simulink model "TestHarness_inf"  (R2016a)
settings: Solver=FixedStepDiscrete  StartTime=0.0  StopTime=120  FixedStep=0.001  RelTol=1e-3
undefined symbols (not defined in the file or in its folder): sigbuilder_block
libraries: simulink x8, gate_V32_2016a_Lib_inf x1, realtime_pacer_lib x1
settings Model: Creator=oelkawok  LastModifiedBy=oelkawok
settings DataIOCC: SaveFormat=StructureWithTime
settings OptimizationCC: DefaultParameterBehavior=Inlined  StateBitsets=on  DataBitsets=on  LifeSpan=1  MaxStackSize=64
settings DebuggingCC: ConsistencyChecking=error  ArrayBoundsChecking=error  SignalInfNanChecking=error  SignalRangeChecking=error  ReadBeforeWriteMsg=EnableAllAsError  WriteAfterWriteMsg=EnableAllAsError  WriteAfterReadMsg=EnableAllAsError  AlgebraicLoopMsg=error  ArtificialAlgebraicLoopMsg=error  BlockPriorityViolationMsg=error  MinStepSizeMsg=error  MultiTaskCondExecSysMsg=none  MultiTaskRateTransMsg=warning  CheckMatrixSingularityMsg=error  ParameterUnderflowMsg=error  ParameterPrecisionLossMsg=error  UnderSpecifiedDataTypeMsg=warning  UnnecessaryDatatypeConvMsg=warning  VectorMatrixConversionMsg=error  SignalLabelMismatchMsg=error  UnconnectedInputMsg=error  UnconnectedOutputMsg=error  UnconnectedLineMsg=error  SFcnCompatibilityMsg=error  UniqueDataStoreMsg=error  BusObjectLabelMismatch=error  RootOutportRequireBusObject=error  ModelReferenceIOMsg=error  ModelReferenceVersionMismatchMessage=error  ModelReferenceIOMismatchMessage=error  ModelReferenceDataLoggingMessage=error  StrictBusMsg=ErrorOnBusTreatedAsVector  SFUnusedDataAndEventsDiag=error  SFUnexpectedBacktrackingDiag=error  SFInvalidInputDataAccessInChartInitDiag=error  SFNoUnconditionalDefaultTransitionDiag=error  SFTransitionOutsideNaturalParentDiag=error  SFUndirectedBroadcastEventsDiag=error  SFTransitionActionBeforeConditionDiag=error  SFOutputUsedAsStateInMooreChartDiag=warning
settings HardwareCC: ProdLongLongMode=on
settings SFSimCC: SimCustomHeaderCode=|
  | //#include "C:\Users\omaro\Desktop\Gate_Model\Arduino\Arduino.h"
  | //#include "Keypad\Keypad.h"
settings RTWCC: SystemTargetFile=ert.tlc  TemplateMakefile=ert_default_tmf  GenerateReport=on  RTWUseSimCustomCode=on  Toolchain=Arduino AVR v1.6.1  BuildConfiguration=Faster Runs  LaunchReport=on
settings RTWCC: CustomHeaderCode=|
  | //#include "C:\Users\omaro\Desktop\Gate_Model\Arduino\Arduino.h"
  | //#include "Keypad\Keypad.h"
settings CodeAppCC: ForceParamTrailComments=on  OperatorAnnotations=on  MATLABSourceComments=on
settings ERTTargetCC: ERTCustomFileBanners=off  SupportContinuousTime=on  SupportNonInlinedSFcns=on  SupportVariableSizeSignals=on  ModelStepFunctionPrototypeControlCompliant=off  RootIOFormat=Structure reference  ERTCustomFileTemplate=example_file_process.tlc

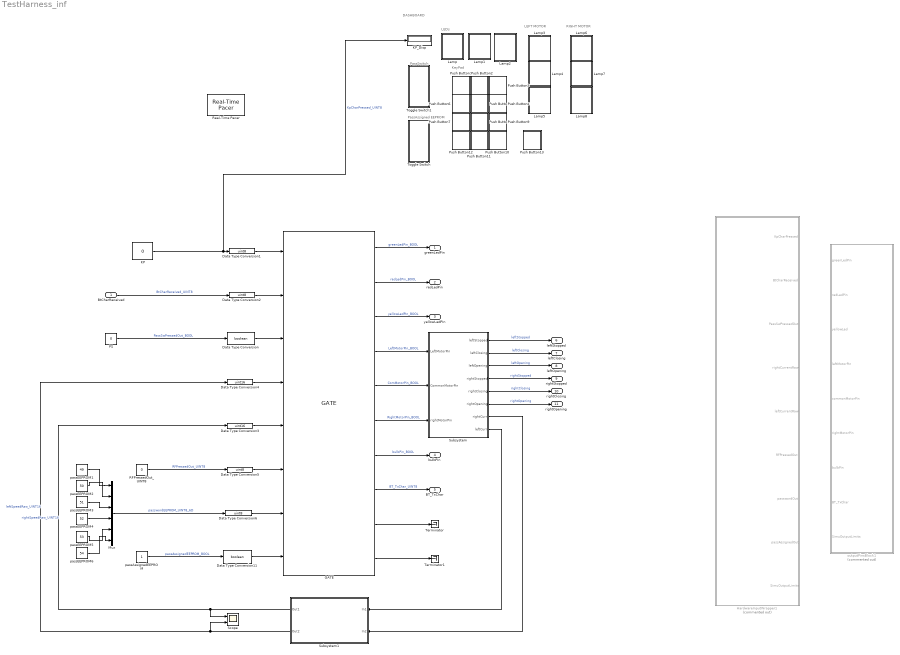
== system TestHarness_inf ==
  note: PassSwitch
  note: PassAssigned
      | EEPROM
  note: KeyPad
  note: DASHBOARD
  note: LEDs
  note: LEFT MOTOR
  note: RIGHT MOTOR
  "BtCharReceived " : Inport
  Data Type Conversion : DataTypeConversion  OutDataTypeStr=boolean
  Data Type Conversion{1..2,5..6} (4 alike) : DataTypeConversion  OutDataTypeStr=uint8
  Data Type Conversion11 : DataTypeConversion  OutDataTypeStr=boolean
  Data Type Conversion3 : DataTypeConversion  OutDataTypeStr=uint16
  Data Type Conversion4 : DataTypeConversion  OutDataTypeStr=uint16
  GATE : gate_V32_2016a_Lib_inf/GATE  VariantControl=Variant1  OverrideUsingVariant=VariantB
  HardwareInputWrapper1 : Subsystem  Commented=on  Variant=on  VariantControl=Variant1  OverrideUsingVariant=MIL_VariantB
  KP : Constant  Value=0  SampleTime=-1
  KP_Disp : Display
      settings: Format=decimal (Stored Integer)
  Lamp : Subsystem  DialogController=hmiCreateDDGDialog  IsWebBlock=on  mask(MWDashboardBlock){webBlockId=dfeecf7f-6466-4b86-8ae3-9a987e31c94e, webBlockType=lamp, ShowInLibBrowser=on, ShowInitialText=on, HMISrcModelName=TestHarness_inf}
      mask prompts: webBlockType "WebBlock Type"; HMISrcModelName "Source Model"
      mask Help: helpview([docroot '/mapfiles/simulink.map'], 'hmi_lamp')
      mask Display:
      | if strcmp(get_param(bdroot(gcb),'BlockDiagramType'), 'library')
      |     image([(matlabroot) '/toolbox/simulink/hmi/icons/Lamp.png']);
      | end
  Lamp1 : Subsystem  DialogController=hmiCreateDDGDialog  IsWebBlock=on  mask(MWDashboardBlock){webBlockId=77b78638-3987-4679-812f-402e2e1d2cbf, webBlockType=lamp, ShowInLibBrowser=on, ShowInitialText=on, HMISrcModelName=TestHarness_inf}
      mask prompts: webBlockType "WebBlock Type"; HMISrcModelName "Source Model"
      mask Help: helpview([docroot '/mapfiles/simulink.map'], 'hmi_lamp')
      mask Display:
      | if strcmp(get_param(bdroot(gcb),'BlockDiagramType'), 'library')
      |     image([(matlabroot) '/toolbox/simulink/hmi/icons/Lamp.png']);
      | end
  Lamp2 : Subsystem  DialogController=hmiCreateDDGDialog  IsWebBlock=on  mask(MWDashboardBlock){webBlockId=16a95052-e057-46e6-b8f2-472c9f701692, webBlockType=lamp, ShowInLibBrowser=on, ShowInitialText=on, HMISrcModelName=TestHarness_inf}
      mask prompts: webBlockType "WebBlock Type"; HMISrcModelName "Source Model"
      mask Help: helpview([docroot '/mapfiles/simulink.map'], 'hmi_lamp')
      mask Display:
      | if strcmp(get_param(bdroot(gcb),'BlockDiagramType'), 'library')
      |     image([(matlabroot) '/toolbox/simulink/hmi/icons/Lamp.png']);
      | end
  Lamp3 : Subsystem  DialogController=hmiCreateDDGDialog  IsWebBlock=on  mask(MWDashboardBlock){webBlockId=c8f1e96f-8ae5-4205-bff4-16b6e81f721f, webBlockType=lamp, ShowInLibBrowser=on, ShowInitialText=on, HMISrcModelName=TestHarness_inf}
      mask prompts: webBlockType "WebBlock Type"; HMISrcModelName "Source Model"
      mask Help: helpview([docroot '/mapfiles/simulink.map'], 'hmi_lamp')
      mask Display:
      | if strcmp(get_param(bdroot(gcb),'BlockDiagramType'), 'library')
      |     image([(matlabroot) '/toolbox/simulink/hmi/icons/Lamp.png']);
      | end
  Lamp4 : Subsystem  DialogController=hmiCreateDDGDialog  IsWebBlock=on  mask(MWDashboardBlock){webBlockId=9178fee7-4670-4670-9e55-d638f178c070, webBlockType=lamp, ShowInLibBrowser=on, ShowInitialText=on, HMISrcModelName=TestHarness_inf}
      mask prompts: webBlockType "WebBlock Type"; HMISrcModelName "Source Model"
      mask Help: helpview([docroot '/mapfiles/simulink.map'], 'hmi_lamp')
      mask Display:
      | if strcmp(get_param(bdroot(gcb),'BlockDiagramType'), 'library')
      |     image([(matlabroot) '/toolbox/simulink/hmi/icons/Lamp.png']);
      | end
  Lamp5 : Subsystem  DialogController=hmiCreateDDGDialog  IsWebBlock=on  mask(MWDashboardBlock){webBlockId=d643292f-cf1e-4cb5-b48c-b958215c0bd7, webBlockType=lamp, ShowInLibBrowser=on, ShowInitialText=on, HMISrcModelName=TestHarness_inf}
      mask prompts: webBlockType "WebBlock Type"; HMISrcModelName "Source Model"
      mask Help: helpview([docroot '/mapfiles/simulink.map'], 'hmi_lamp')
      mask Display:
      | if strcmp(get_param(bdroot(gcb),'BlockDiagramType'), 'library')
      |     image([(matlabroot) '/toolbox/simulink/hmi/icons/Lamp.png']);
      | end
  Lamp6 : Subsystem  DialogController=hmiCreateDDGDialog  IsWebBlock=on  mask(MWDashboardBlock){webBlockId=6bb08dff-de60-4262-bf7f-c7f88bbee20a, webBlockType=lamp, ShowInLibBrowser=on, ShowInitialText=on, HMISrcModelName=TestHarness_inf}
      mask prompts: webBlockType "WebBlock Type"; HMISrcModelName "Source Model"
      mask Help: helpview([docroot '/mapfiles/simulink.map'], 'hmi_lamp')
      mask Display:
      | if strcmp(get_param(bdroot(gcb),'BlockDiagramType'), 'library')
      |     image([(matlabroot) '/toolbox/simulink/hmi/icons/Lamp.png']);
      | end
  Lamp7 : Subsystem  DialogController=hmiCreateDDGDialog  IsWebBlock=on  mask(MWDashboardBlock){webBlockId=b5666a4d-f33d-4bf5-ba64-e318e21455ef, webBlockType=lamp, ShowInLibBrowser=on, ShowInitialText=on, HMISrcModelName=TestHarness_inf}
      mask prompts: webBlockType "WebBlock Type"; HMISrcModelName "Source Model"
      mask Help: helpview([docroot '/mapfiles/simulink.map'], 'hmi_lamp')
      mask Display:
      | if strcmp(get_param(bdroot(gcb),'BlockDiagramType'), 'library')
      |     image([(matlabroot) '/toolbox/simulink/hmi/icons/Lamp.png']);
      | end
  Lamp8 : Subsystem  DialogController=hmiCreateDDGDialog  IsWebBlock=on  mask(MWDashboardBlock){webBlockId=b7bdd2c5-6b26-4275-9419-c5f65add7689, webBlockType=lamp, ShowInLibBrowser=on, ShowInitialText=on, HMISrcModelName=TestHarness_inf}
      mask prompts: webBlockType "WebBlock Type"; HMISrcModelName "Source Model"
      mask Help: helpview([docroot '/mapfiles/simulink.map'], 'hmi_lamp')
      mask Display:
      | if strcmp(get_param(bdroot(gcb),'BlockDiagramType'), 'library')
      |     image([(matlabroot) '/toolbox/simulink/hmi/icons/Lamp.png']);
      | end
  Mux : Mux  Inputs=6
  PS : Constant  Value=0
  Push Button1 : Subsystem  DialogController=hmiCreateDDGDialog  IsWebBlock=on  mask(MWDashboardBlock){webBlockId=efb186e0-7ac7-42d4-9545-c8cda7e6d6ff, webBlockType=pushbutton, ShowInLibBrwoser=on, ShowInitialText=on, HMISrcModelName=TestHarness_inf}
      mask prompts: webBlockId "WebBlock Id"; webBlockType "WebBlock Type"; HMISrcModelName "Source Model"
      mask Help: helpview([docroot '/mapfiles/simulink.map'], 'hmi_push_button')
      mask Display:
      | model = bdroot(gcb);
      | if strcmp(get_param(model,'BlockDiagramType'), 'library')
      |     image([(matlabroot) '/toolbox/simulink/hmi/icons/PushButton.png']);
      | end
  Push Button10 : Subsystem  DialogController=hmiCreateDDGDialog  IsWebBlock=on  mask(MWDashboardBlock){webBlockId=a72d1c9d-87a6-4091-a506-e1108b8799d8, webBlockType=pushbutton, ShowInLibBrwoser=on, ShowInitialText=on, HMISrcModelName=TestHarness_inf}
      mask prompts: webBlockId "WebBlock Id"; webBlockType "WebBlock Type"; HMISrcModelName "Source Model"
      mask Help: helpview([docroot '/mapfiles/simulink.map'], 'hmi_push_button')
      mask Display:
      | model = bdroot(gcb);
      | if strcmp(get_param(model,'BlockDiagramType'), 'library')
      |     image([(matlabroot) '/toolbox/simulink/hmi/icons/PushButton.png']);
      | end
  Push Button11 : Subsystem  DialogController=hmiCreateDDGDialog  IsWebBlock=on  mask(MWDashboardBlock){webBlockId=d494c8aa-0474-4b50-982c-5f796114d678, webBlockType=pushbutton, ShowInLibBrwoser=on, ShowInitialText=on, HMISrcModelName=TestHarness_inf}
      mask prompts: webBlockId "WebBlock Id"; webBlockType "WebBlock Type"; HMISrcModelName "Source Model"
      mask Help: helpview([docroot '/mapfiles/simulink.map'], 'hmi_push_button')
      mask Display:
      | model = bdroot(gcb);
      | if strcmp(get_param(model,'BlockDiagramType'), 'library')
      |     image([(matlabroot) '/toolbox/simulink/hmi/icons/PushButton.png']);
      | end
  Push Button12 : Subsystem  DialogController=hmiCreateDDGDialog  IsWebBlock=on  mask(MWDashboardBlock){webBlockId=f33ddd79-ca68-49fe-b4b6-aee4b307d83a, webBlockType=pushbutton, ShowInLibBrwoser=on, ShowInitialText=on, HMISrcModelName=TestHarness_inf}
      mask prompts: webBlockId "WebBlock Id"; webBlockType "WebBlock Type"; HMISrcModelName "Source Model"
      mask Help: helpview([docroot '/mapfiles/simulink.map'], 'hmi_push_button')
      mask Display:
      | model = bdroot(gcb);
      | if strcmp(get_param(model,'BlockDiagramType'), 'library')
      |     image([(matlabroot) '/toolbox/simulink/hmi/icons/PushButton.png']);
      | end
  Push Button13 : Subsystem  DialogController=hmiCreateDDGDialog  IsWebBlock=on  mask(MWDashboardBlock){webBlockId=e5fe72a6-0ccb-4320-95ea-2ffa7a798bea, webBlockType=pushbutton, ShowInLibBrwoser=on, ShowInitialText=on, HMISrcModelName=TestHarness_inf}
      mask prompts: webBlockId "WebBlock Id"; webBlockType "WebBlock Type"; HMISrcModelName "Source Model"
      mask Help: helpview([docroot '/mapfiles/simulink.map'], 'hmi_push_button')
      mask Display:
      | model = bdroot(gcb);
      | if strcmp(get_param(model,'BlockDiagramType'), 'library')
      |     image([(matlabroot) '/toolbox/simulink/hmi/icons/PushButton.png']);
      | end
  Push Button2 : Subsystem  DialogController=hmiCreateDDGDialog  IsWebBlock=on  mask(MWDashboardBlock){webBlockId=fffe111a-15fb-434e-8e7d-6c74e690b8ec, webBlockType=pushbutton, ShowInLibBrwoser=on, ShowInitialText=on, HMISrcModelName=TestHarness_inf}
      mask prompts: webBlockId "WebBlock Id"; webBlockType "WebBlock Type"; HMISrcModelName "Source Model"
      mask Help: helpview([docroot '/mapfiles/simulink.map'], 'hmi_push_button')
      mask Display:
      | model = bdroot(gcb);
      | if strcmp(get_param(model,'BlockDiagramType'), 'library')
      |     image([(matlabroot) '/toolbox/simulink/hmi/icons/PushButton.png']);
      | end
  Push Button3 : Subsystem  DialogController=hmiCreateDDGDialog  IsWebBlock=on  mask(MWDashboardBlock){webBlockId=7a7e2e11-9f3b-43a3-8fe5-ea89bc2462e2, webBlockType=pushbutton, ShowInLibBrwoser=on, ShowInitialText=on, HMISrcModelName=TestHarness_inf}
      mask prompts: webBlockId "WebBlock Id"; webBlockType "WebBlock Type"; HMISrcModelName "Source Model"
      mask Help: helpview([docroot '/mapfiles/simulink.map'], 'hmi_push_button')
      mask Display:
      | model = bdroot(gcb);
      | if strcmp(get_param(model,'BlockDiagramType'), 'library')
      |     image([(matlabroot) '/toolbox/simulink/hmi/icons/PushButton.png']);
      | end
  Push Button4 : Subsystem  DialogController=hmiCreateDDGDialog  IsWebBlock=on  mask(MWDashboardBlock){webBlockId=4e418dfc-3c1d-4c60-a82e-ca2e05426c13, webBlockType=pushbutton, ShowInLibBrwoser=on, ShowInitialText=on, HMISrcModelName=TestHarness_inf}
      mask prompts: webBlockId "WebBlock Id"; webBlockType "WebBlock Type"; HMISrcModelName "Source Model"
      mask Help: helpview([docroot '/mapfiles/simulink.map'], 'hmi_push_button')
      mask Display:
      | model = bdroot(gcb);
      | if strcmp(get_param(model,'BlockDiagramType'), 'library')
      |     image([(matlabroot) '/toolbox/simulink/hmi/icons/PushButton.png']);
      | end
  Push Button5 : Subsystem  DialogController=hmiCreateDDGDialog  IsWebBlock=on  mask(MWDashboardBlock){webBlockId=b94535ab-6966-44e9-8d7e-8f23ab203339, webBlockType=pushbutton, ShowInLibBrwoser=on, ShowInitialText=on, HMISrcModelName=TestHarness_inf}
      mask prompts: webBlockId "WebBlock Id"; webBlockType "WebBlock Type"; HMISrcModelName "Source Model"
      mask Help: helpview([docroot '/mapfiles/simulink.map'], 'hmi_push_button')
      mask Display:
      | model = bdroot(gcb);
      | if strcmp(get_param(model,'BlockDiagramType'), 'library')
      |     image([(matlabroot) '/toolbox/simulink/hmi/icons/PushButton.png']);
      | end
  Push Button6 : Subsystem  DialogController=hmiCreateDDGDialog  IsWebBlock=on  mask(MWDashboardBlock){webBlockId=823970b4-3e8f-4edd-8037-ba652d66eccb, webBlockType=pushbutton, ShowInLibBrwoser=on, ShowInitialText=on, HMISrcModelName=TestHarness_inf}
      mask prompts: webBlockId "WebBlock Id"; webBlockType "WebBlock Type"; HMISrcModelName "Source Model"
      mask Help: helpview([docroot '/mapfiles/simulink.map'], 'hmi_push_button')
      mask Display:
      | model = bdroot(gcb);
      | if strcmp(get_param(model,'BlockDiagramType'), 'library')
      |     image([(matlabroot) '/toolbox/simulink/hmi/icons/PushButton.png']);
      | end
  Push Button7 : Subsystem  DialogController=hmiCreateDDGDialog  IsWebBlock=on  mask(MWDashboardBlock){webBlockId=b1b2ae4e-6aee-480e-a413-dcdbe3967c1c, webBlockType=pushbutton, ShowInLibBrwoser=on, ShowInitialText=on, HMISrcModelName=TestHarness_inf}
      mask prompts: webBlockId "WebBlock Id"; webBlockType "WebBlock Type"; HMISrcModelName "Source Model"
      mask Help: helpview([docroot '/mapfiles/simulink.map'], 'hmi_push_button')
      mask Display:
      | model = bdroot(gcb);
      | if strcmp(get_param(model,'BlockDiagramType'), 'library')
      |     image([(matlabroot) '/toolbox/simulink/hmi/icons/PushButton.png']);
      | end
  Push Button8 : Subsystem  DialogController=hmiCreateDDGDialog  IsWebBlock=on  mask(MWDashboardBlock){webBlockId=3cad68d3-fab3-48c6-a191-870e657ebab3, webBlockType=pushbutton, ShowInLibBrwoser=on, ShowInitialText=on, HMISrcModelName=TestHarness_inf}
      mask prompts: webBlockId "WebBlock Id"; webBlockType "WebBlock Type"; HMISrcModelName "Source Model"
      mask Help: helpview([docroot '/mapfiles/simulink.map'], 'hmi_push_button')
      mask Display:
      | model = bdroot(gcb);
      | if strcmp(get_param(model,'BlockDiagramType'), 'library')
      |     image([(matlabroot) '/toolbox/simulink/hmi/icons/PushButton.png']);
      | end
  Push Button9 : Subsystem  DialogController=hmiCreateDDGDialog  IsWebBlock=on  mask(MWDashboardBlock){webBlockId=86647f27-4547-4003-afd8-e3dfd8bf4d4c, webBlockType=pushbutton, ShowInLibBrwoser=on, ShowInitialText=on, HMISrcModelName=TestHarness_inf}
      mask prompts: webBlockId "WebBlock Id"; webBlockType "WebBlock Type"; HMISrcModelName "Source Model"
      mask Help: helpview([docroot '/mapfiles/simulink.map'], 'hmi_push_button')
      mask Display:
      | model = bdroot(gcb);
      | if strcmp(get_param(model,'BlockDiagramType'), 'library')
      |     image([(matlabroot) '/toolbox/simulink/hmi/icons/PushButton.png']);
      | end
  RFPressedOut_UINT8 : Constant  Value=0
  Real-Time Pacer : realtime_pacer_lib/Real-Time Pacer  simTimePerRealTime=1
  Scope : Scope  NumInputPorts=2
  Subsystem : Subsystem
  Subsystem1 : Subsystem
  Terminator : Terminator
  Terminator1 : Terminator
  Toggle Switch : Subsystem  DialogController=hmiCreateDDGDialog  IsWebBlock=on  mask(MWDashboardBlock){webBlockId=d58e30f4-5a6a-4cfe-92f1-adc9eee3422c, webBlockType=toggleswitch, ShowInLibBrowser=on, ShowInitialText=on, HMISrcModelName=TestHarness_inf}
      mask prompts: webBlockId "WebBlock Id"; webBlockType "WebBlock Type"; HMISrcModelName "Source Model"
      mask Help: helpview([docroot '/mapfiles/simulink.map'], 'hmi_toggle_switch')
      mask Display:
      | if strcmp(get_param(bdroot(gcb),'BlockDiagramType'), 'library')
      |     image([(matlabroot) '/toolbox/simulink/hmi/icons/ToggleSwitch.png']);
      | end
  Toggle Switch1 : Subsystem  DialogController=hmiCreateDDGDialog  IsWebBlock=on  mask(MWDashboardBlock){webBlockId=f86417cf-80a7-4500-9ed8-29fb0c2a7cd2, webBlockType=toggleswitch, ShowInLibBrowser=on, ShowInitialText=on, HMISrcModelName=TestHarness_inf}
      mask prompts: webBlockId "WebBlock Id"; webBlockType "WebBlock Type"; HMISrcModelName "Source Model"
      mask Help: helpview([docroot '/mapfiles/simulink.map'], 'hmi_toggle_switch')
      mask Display:
      | if strcmp(get_param(bdroot(gcb),'BlockDiagramType'), 'library')
      |     image([(matlabroot) '/toolbox/simulink/hmi/icons/ToggleSwitch.png']);
      | end
  outputPinsBlock1 : Subsystem  Commented=on  Variant=on  VariantControl=Variant1  OverrideUsingVariant=MIL_ALL_Variants
  passAssignedEEPROM : Constant
  passEEPROM1 : Constant  Value=49
  passEEPROM2 : Constant  Value=50
  passEEPROM3 : Constant  Value=51
  passEEPROM4 : Constant  Value=52
  passEEPROM5 : Constant  Value=53
  passEEPROM6 : Constant  Value=54
  " greenLedPin" : Outport
  " redLedPin" : Outport  Port=2
  " yellowLedPin" : Outport  Port=3
  " bulbPin" : Outport  Port=4
  " BT_TxChar" : Outport  Port=5
  leftStopped : Outport  Port=6
  leftClosing : Outport  Port=7
  leftOpening : Outport  Port=8
  rightStopped : Outport  Port=9
  rightClosing : Outport  Port=10
  rightOpening : Outport  Port=11
  wires:
  GATE/1 -> " greenLedPin"/1  "greenLedPin_BOOL"
  GATE/2 -> " redLedPin"/1  "redLedPin_BOOL"
  GATE/3 -> " yellowLedPin"/1  "yellowLedPin_BOOL"
  GATE/4 -> Subsystem/1(LeftMotorPin)  "LeftMotorPin_BOOL"
  GATE/5 -> Subsystem/2(CommonMotorPin)  "ComMotorPin_BOOL"
  GATE/6 -> Subsystem/3(rightMotorPin)  "RightMotorPin_BOOL"
  GATE/7 -> " bulbPin"/1  "bulbPin_BOOL"
  GATE/8 -> " BT_TxChar"/1  "BT_TxChar_UINT8"
  "BtCharReceived "/1 -> Data Type Conversion2/1  "BtCharReceived_UINT8"
  PS/1 -> Data Type Conversion/1  "PassSwPressedOut_BOOL"
  RFPressedOut_UINT8/1 -> Data Type Conversion5/1  "RFPressedOut_UINT8"
  KP/1 -> KP_Disp/1, Data Type Conversion1/1  "KpCharPressed_UINT8"
  Mux/1 -> Data Type Conversion6/1  "passwordEEPROM_UINT8_6D"
  passAssignedEEPROM/1 -> Data Type Conversion11/1  "passAssignedEEPROM_BOOL"
  Data Type Conversion1/1 -> GATE/1
  Data Type Conversion2/1 -> GATE/2
  Data Type Conversion/1 -> GATE/3
  Data Type Conversion4/1 -> GATE/4
  Data Type Conversion3/1 -> GATE/5
  Data Type Conversion5/1 -> GATE/6
  Data Type Conversion6/1 -> GATE/7
  Data Type Conversion11/1 -> GATE/8
  GATE/9 -> Terminator/1
  GATE/10 -> Terminator1/1
  Subsystem/1(leftStopped) -> leftStopped/1  "leftStopped"
  Subsystem/2(leftClosing) -> leftClosing/1  "leftClosing"
  Subsystem/4(rightStopped) -> rightStopped/1  "rightStopped"
  Subsystem/5(rightClosing) -> rightClosing/1  "rightClosing"
  Subsystem/6(rightOpening) -> rightOpening/1  "rightOpening"
  Subsystem/3(leftOpening) -> leftOpening/1  "leftOpening"
  Subsystem/8(leftCurr) -> Subsystem1/1(In1)
  Subsystem/7(rightCurr) -> Subsystem1/2(In2)
  Subsystem1/1(Out1) -> Scope/1, Data Type Conversion3/1  "rightSpeedRaw_UINT16"
  Subsystem1/2(Out2) -> Scope/2, Data Type Conversion4/1  "leftSpeedRaw_UINT16"
  passEEPROM4/1 -> Mux/4
  passEEPROM3/1 -> Mux/3
  passEEPROM5/1 -> Mux/5
  passEEPROM2/1 -> Mux/2
  passEEPROM6/1 -> Mux/6
  passEEPROM1/1 -> Mux/1

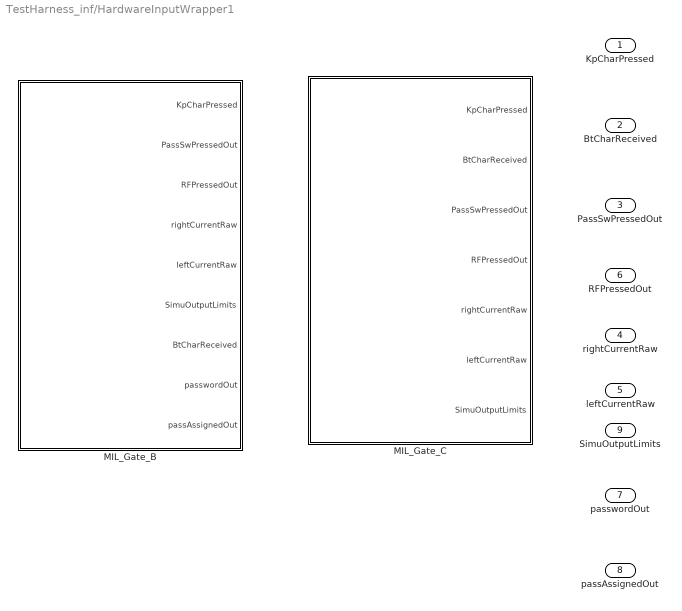
== system TestHarness_inf/HardwareInputWrapper1 ==
  MIL_Gate_B : Subsystem  VariantControl=MIL_VariantB
  MIL_Gate_C : Subsystem  VariantControl=MIL_VariantC
  KpCharPressed : Outport
  BtCharReceived : Outport  Port=2
  PassSwPressedOut : Outport  Port=3
  rightCurrentRaw : Outport  Port=4
  leftCurrentRaw : Outport  Port=5
  RFPressedOut : Outport  Port=6
  passwordOut : Outport  Port=7
  passAssignedOut : Outport  Port=8
  SimuOutputLimits : Outport  Port=9

== system TestHarness_inf/Lamp ==

== system TestHarness_inf/Lamp1 ==

== system TestHarness_inf/Lamp2 ==

== system TestHarness_inf/Lamp3 ==

== system TestHarness_inf/Lamp4 ==

== system TestHarness_inf/Lamp5 ==

== system TestHarness_inf/Lamp6 ==

== system TestHarness_inf/Lamp7 ==

== system TestHarness_inf/Lamp8 ==

== system TestHarness_inf/Push Button1 ==

== system TestHarness_inf/Push Button10 ==

== system TestHarness_inf/Push Button11 ==

== system TestHarness_inf/Push Button12 ==

== system TestHarness_inf/Push Button13 ==

== system TestHarness_inf/Push Button2 ==

== system TestHarness_inf/Push Button3 ==

== system TestHarness_inf/Push Button4 ==

== system TestHarness_inf/Push Button5 ==

== system TestHarness_inf/Push Button6 ==

== system TestHarness_inf/Push Button7 ==

== system TestHarness_inf/Push Button8 ==

== system TestHarness_inf/Push Button9 ==

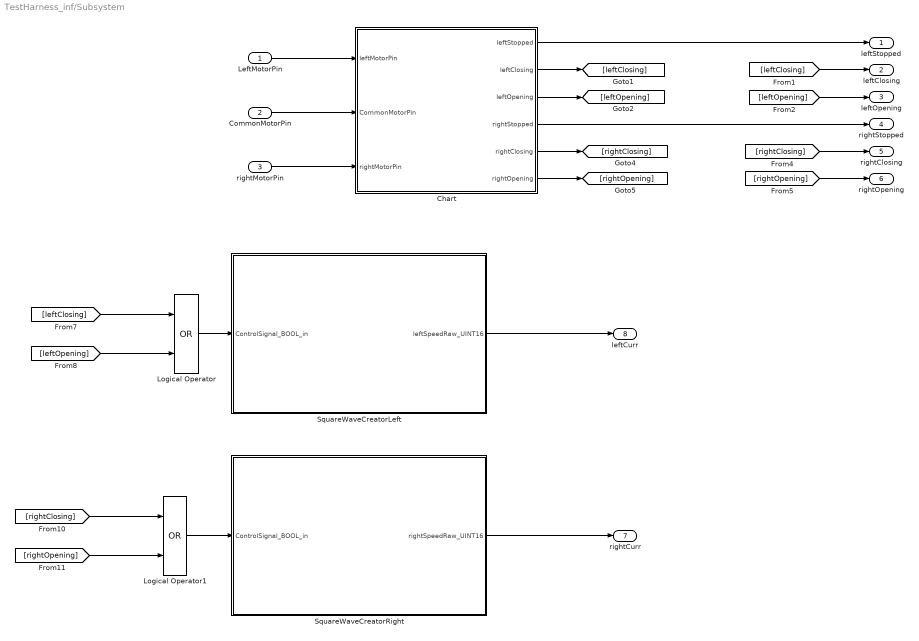
== system TestHarness_inf/Subsystem ==
  LeftMotorPin : Inport
  CommonMotorPin : Inport  Port=2
  rightMotorPin : Inport  Port=3
  Chart : Stateflow chart  TreatAsAtomicUnit=on
  Logical Operator : Logic  Operator=OR  AllPortsSameDT=off  OutDataTypeStr=boolean
  Logical Operator1 : Logic  Operator=OR  AllPortsSameDT=off  OutDataTypeStr=boolean
  SquareWaveCreatorLeft : Stateflow chart  TreatAsAtomicUnit=on
  SquareWaveCreatorRight : Stateflow chart  TreatAsAtomicUnit=on
  leftStopped : Outport
  leftClosing : Outport  Port=2
  leftOpening : Outport  Port=3
  rightStopped : Outport  Port=4
  rightClosing : Outport  Port=5
  rightOpening : Outport  Port=6
  rightCurr : Outport  Port=7
  leftCurr : Outport  Port=8
  wires:
  LeftMotorPin/1 -> Chart/1(leftMotorPin)
  rightMotorPin/1 -> Chart/3(rightMotorPin)
  CommonMotorPin/1 -> Chart/2(CommonMotorPin)
  Chart/1(leftStopped) -> leftStopped/1
  [leftClosing] -> leftClosing/1
  [leftOpening] -> leftOpening/1
  Chart/4(rightStopped) -> rightStopped/1
  [rightClosing] -> rightClosing/1
  [rightOpening] -> rightOpening/1
  Chart/6(rightOpening) -> [rightOpening]
  Chart/5(rightClosing) -> [rightClosing]
  Chart/2(leftClosing) -> [leftClosing]
  Chart/3(leftOpening) -> [leftOpening]
  [leftOpening] -> Logical Operator/2
  [leftClosing] -> Logical Operator/1
  [rightOpening] -> Logical Operator1/2
  [rightClosing] -> Logical Operator1/1
  Logical Operator/1 -> SquareWaveCreatorLeft/1(ControlSignal_BOOL_in)
  SquareWaveCreatorLeft/1(leftSpeedRaw_UINT16) -> leftCurr/1
  Logical Operator1/1 -> SquareWaveCreatorRight/1(ControlSignal_BOOL_in)
  SquareWaveCreatorRight/1(rightSpeedRaw_UINT16) -> rightCurr/1

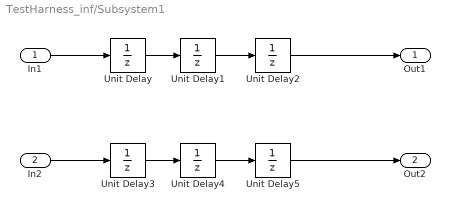
== system TestHarness_inf/Subsystem1 ==
  In1 : Inport
  In2 : Inport  Port=2
  Unit Delay{,1..5} (6 alike) : UnitDelay  SampleTime=-1
  Out1 : Outport
  Out2 : Outport  Port=2
  wires:
  In1/1 -> Unit Delay/1
  Unit Delay/1 -> Unit Delay1/1
  Unit Delay1/1 -> Unit Delay2/1
  Unit Delay2/1 -> Out1/1
  In2/1 -> Unit Delay3/1
  Unit Delay3/1 -> Unit Delay4/1
  Unit Delay4/1 -> Unit Delay5/1
  Unit Delay5/1 -> Out2/1

== system TestHarness_inf/Toggle Switch ==

== system TestHarness_inf/Toggle Switch1 ==

== system TestHarness_inf/outputPinsBlock1 ==
  greenLedPin : Inport
  redLedPin : Inport  Port=2
  yellowLed : Inport  Port=3
  leftMotorPin : Inport  Port=4
  commonMotorPin : Inport  Port=5
  rightMotorPin : Inport  Port=6
  bulbPin : Inport  Port=7
  BT_TxChar : Inport  Port=8
  SimuOutputLimits : Inport  Port=9
  MIL : Subsystem  Variant=on  VariantControl=MIL_ALL_Variants  OverrideUsingVariant=VisualOutputs
